simulink model "CCMSysServ"  (R2022b)
settings: Solver=FixedStepDiscrete  StartTime=0.0  StopTime=10.0  FixedStep=0.05  RelTol=1e-3
model workspace (MAT data in the model file):
  NvMBlockCarCfgData : Simulink.Signal (value not decoded)
  NvMBlockCarCfgDataInit : Simulink.Parameter (value not decoded)
  NvMBlockDidDataD005 : Simulink.Signal (value not decoded)
  NvMBlockDidDataD005Init : Simulink.Parameter (value not decoded)
  NvMBlockDidDataD09A : Simulink.Signal (value not decoded)
  NvMBlockDidDataD09AInit : Simulink.Parameter (value not decoded)
  NvMBlockDidDataE103 : Simulink.Signal (value not decoded)
  NvMBlockDidDataE103Init : Simulink.Parameter (value not decoded)
  NvMBlockDidDataF190 : Simulink.Signal (value not decoded)
  NvMBlockDidDataF190Init : Simulink.Parameter (value not decoded)
  NvMBlockDidDataF1AB : Simulink.Signal (value not decoded)
  NvMBlockDidDataF1ABInit : Simulink.Parameter (value not decoded)
symbols that are objects in the model workspace (value not decoded): NvMBlockCarCfgDataInit, NvMBlockDidDataD005Init, NvMBlockDidDataD09AInit, NvMBlockDidDataE103Init, NvMBlockDidDataF190Init, NvMBlockDidDataF1ABInit
(part 2 of 4)

== system CCMSysServ/CCMSysServ_Init ==
  Event Listener : EventListener  EventName=reset
  PA_NvMBlockCarCfgDataInit : Constant  Value=NvMBlockCarCfgDataInit
  PA_NvMBlockDidDataD005Init : Constant  Value=NvMBlockDidDataD005Init
  PA_NvMBlockDidDataD09AInit : Constant  Value=NvMBlockDidDataD09AInit
  PA_NvMBlockDidDataE103Init : Constant  Value=NvMBlockDidDataE103Init
  PA_NvMBlockDidDataF190Init : Constant  Value=NvMBlockDidDataF190Init
  PA_NvMBlockDidDataF1ABInit : Constant  Value=NvMBlockDidDataF1ABInit
  Terminator_{1..6} (6 alike) : Terminator
  wires:
  PA_NvMBlockCarCfgDataInit/1 -> Terminator_1/1
  PA_NvMBlockDidDataD005Init/1 -> Terminator_2/1
  PA_NvMBlockDidDataD09AInit/1 -> Terminator_3/1
  PA_NvMBlockDidDataE103Init/1 -> Terminator_4/1
  PA_NvMBlockDidDataF190Init/1 -> Terminator_5/1
  PA_NvMBlockDidDataF1ABInit/1 -> Terminator_6/1
